simulink model "souk_dual_pipeline_4x2"  (R2021a)
settings: Solver=VariableStepAuto  StartTime=0.0  StopTime=10.0  RelTol=1e-3
undefined symbols (not defined in the file): datetime, posixtime
libraries: xbsIndex_r4 x14695, casper_library_misc x154, casper_library_delays x68, casper_library_reorder x64, casper_library_multipliers x16, xps_library x9
(part 2 of 164)

== system souk_dual_pipeline_4x2/p0 ==
  din_i : Inport
  din_q : Inport  Port=2
  sync_in : Inport  Port=3
  From1 : From  Commented=on  GotoTag=mrst
  Terminator{,1..3} (4 alike) : Terminator
  acc0 : Subsystem  mask{nchan_serial_bits=8, nwindow_bits=11}
      mask prompts: nchan_serial_bits "Number of serial channels (2^?)"; nwindow_bits "Window points (2^?)"
  acc1 : Subsystem  mask{nchan_serial_bits=8, nwindow_bits=11}
      mask prompts: nchan_serial_bits "Number of serial channels (2^?)"; nwindow_bits "Window points (2^?)"
  autocorr : Subsystem  Commented=on  mask{nchan_bits=12-4, npol_bits=0}
      mask prompts: nchan_bits "Number of *SERIAL* channels (2^?)"; npol_bits "Number of pols (2^?)"
  chan_select : Subsystem  mask{serial_chan_bits=8}
      mask prompts: serial_chan_bits "Number of serial channels (2^?)"
  cordic_gen : Subsystem  Commented=on  mask{n_cordic=1, n_parallel=8}
      mask prompts: n_cordic "Number of independent generators?"; n_parallel "Number of parallel samples?"
  edge_detect : casper_library_misc/edge_detect  edge=Rising  polarity=Active High  x_in=[0 0.08 0.08 0.16 0.16 0.24 0.24 0.32 0.32 0.4]  y_in="[-1 -1  1  1  1  1  1  1  1  1]"  x_out=[0.6 0.68 0.68 0.76 0.76 0.84 0.84 0.92 0.92 1]  y_out="[-1 -1  1  1 -1 -1 -1 -1 -1 -1]"
  input : Subsystem
  lut_gen : Subsystem  Commented=on  mask{n_generator=1, n_parallel=8, n_sample_bits=12}
      mask prompts: n_generator "Number of independent generators?"; n_parallel "Number of parallel samples?"; n_sample_bits "Number of samples total (2^?)"
  mix : Subsystem  mask{serial_chan_bits=8}
      mask prompts: serial_chan_bits "Number of serial channels (2^?)"
  output : Subsystem  Commented=on
  pfb : Subsystem
  pfbtvg : Subsystem  Commented=through  mask{serial_chan_bits=8}
      mask prompts: serial_chan_bits "Serial channels (2^?)"
  psb : Subsystem
  pulse_ext : Subsystem  AncestorBlock=casper_library_misc/pulse_ext  mask(pulse_ext){pulse_len=2^25}
      mask prompts: pulse_len "Length of Pulse"
      mask help:
      | Extends a boolean signal to be high for the specified number of clocks after
      | the last high input.
      mask init:
      | bits = ceil(log2(pulse_len+1));
      mask Help: eval('xlWeb(''http://casper.berkeley.edu/wiki/Pulse_ext'')')
  sync : Subsystem  mask{sync_lsb_bits=14, sync_period=307200000}
      mask prompts: sync_lsb_bits "Allowed sync granularity (2^?)"; sync_period "Expected_sync_period"
  synth_input_reorder : Subsystem  mask{serial_chan_bits=8}
      mask prompts: serial_chan_bits "Number of serial channels (2^?)"
  synth_offset_input_reorder : Subsystem  mask{serial_chan_bits=8}
      mask prompts: serial_chan_bits "Number of serial channels (2^?)"
  zoom_acc : Subsystem  Commented=on  mask{nchan_serial_bits=10, downsample_factor=256}
      mask prompts: nchan_serial_bits "Number of serial channels (2^?)"; downsample_factor "Downsample factor?"
  zoom_fft : Subsystem  Commented=on
  dout : Outport
  pps_led : Outport  Port=2
  wires:
  synth_input_reorder/2(dout) -> psb/2(din0)
  chan_select/1(sync_out) -> mix/1(sync), zoom_fft/1(sync)
  zoom_fft/1(sync_out) -> zoom_acc/1(sync)
  synth_offset_input_reorder/2(dout) -> psb/3(din1)
  lut_gen/1(dout) -> output/2(din1)
  cordic_gen/1(dout) -> output/1(din0)
  [sync_gen] -> input/1(sync)
  sync/4(sync_out) -> [sync_gen]
  [mrst] -> mix/3(rst)
  pfbtvg/2(dout) -> chan_select/2(din), autocorr/2(din)
  input/2(dout) -> pfb/2(din)
  sync/1(arm_noise) -> Terminator/1
  chan_select/2(dout) -> mix/2(din), zoom_fft/2(din)
  din_i/1 -> input/2(din_i)
  From1/1 -> autocorr/3(rst)
  sync/7(tt_int_out) -> Terminator2/1
  sync/8(err_out) -> Terminator3/1
  mix/2(dout) -> acc1/2(din), acc0/2(din)
  sync/6(timed_sync) -> Terminator1/1
  pfb/2(dout) -> pfbtvg/2(din)
  mix/3(lo_out) -> synth_offset_input_reorder/2(din), synth_input_reorder/2(din)
  synth_input_reorder/1(sync_out) -> psb/1(sync)
  [mrst] -> acc0/4(rst)
  sync/2(tt_sync) -> [sync_time]
  mix/4(lo_sync_out) -> synth_offset_input_reorder/1(sync), synth_input_reorder/1(sync)
  mix/1(sync_out) -> acc1/1(sync), acc0/1(sync)
  din_q/1 -> input/3(din_q)
  pfb/1(sync_out) -> pfbtvg/1(sync)
  [sync_time] -> acc0/3(tt)
  pfbtvg/1(sync_out) -> chan_select/1(sync), autocorr/1(sync)
  sync/5(mrst) -> [mrst]
  psb/2(dout) -> input/4(dac), dout/1, output/3(din2)
  input/1(sync_out) -> pfb/1(sync)
  [mrst] -> acc1/4(rst)
  zoom_fft/2(dout) -> zoom_acc/2(din)
  [sync_time] -> acc1/3(tt)
  sync_in/1 -> edge_detect/1
  edge_detect/1 -> sync/1(sync_in)
  sync/3(ext_sync) -> pulse_ext/1(in)
  pulse_ext/1(out) -> pps_led/1

== system souk_dual_pipeline_4x2/p1 ==
  din_i : Inport
  din_q : Inport  Port=2
  sync_in : Inport  Port=3
  From1 : From  Commented=on  GotoTag=mrst
  Terminator{,1..3} (4 alike) : Terminator
  acc0 : Subsystem  mask{nchan_serial_bits=8, nwindow_bits=11}
      mask prompts: nchan_serial_bits "Number of serial channels (2^?)"; nwindow_bits "Window points (2^?)"
  acc1 : Subsystem  mask{nchan_serial_bits=8, nwindow_bits=11}
      mask prompts: nchan_serial_bits "Number of serial channels (2^?)"; nwindow_bits "Window points (2^?)"
  autocorr : Subsystem  Commented=on  mask{nchan_bits=12-4, npol_bits=0}
      mask prompts: nchan_bits "Number of *SERIAL* channels (2^?)"; npol_bits "Number of pols (2^?)"
  chan_select : Subsystem  mask{serial_chan_bits=8}
      mask prompts: serial_chan_bits "Number of serial channels (2^?)"
  cordic_gen : Subsystem  Commented=on  mask{n_cordic=1, n_parallel=8}
      mask prompts: n_cordic "Number of independent generators?"; n_parallel "Number of parallel samples?"
  edge_detect : casper_library_misc/edge_detect  edge=Rising  polarity=Active High  x_in=[0 0.08 0.08 0.16 0.16 0.24 0.24 0.32 0.32 0.4]  y_in="[-1 -1  1  1  1  1  1  1  1  1]"  x_out=[0.6 0.68 0.68 0.76 0.76 0.84 0.84 0.92 0.92 1]  y_out="[-1 -1  1  1 -1 -1 -1 -1 -1 -1]"
  input : Subsystem
  lut_gen : Subsystem  Commented=on  mask{n_generator=1, n_parallel=8, n_sample_bits=12}
      mask prompts: n_generator "Number of independent generators?"; n_parallel "Number of parallel samples?"; n_sample_bits "Number of samples total (2^?)"
  mix : Subsystem  mask{serial_chan_bits=8}
      mask prompts: serial_chan_bits "Number of serial channels (2^?)"
  output : Subsystem  Commented=on
  pfb : Subsystem
  pfbtvg : Subsystem  Commented=through  mask{serial_chan_bits=8}
      mask prompts: serial_chan_bits "Serial channels (2^?)"
  psb : Subsystem
  pulse_ext : Subsystem  AncestorBlock=casper_library_misc/pulse_ext  mask(pulse_ext){pulse_len=2^25}
      mask prompts: pulse_len "Length of Pulse"
      mask help:
      | Extends a boolean signal to be high for the specified number of clocks after
      | the last high input.
      mask init:
      | bits = ceil(log2(pulse_len+1));
      mask Help: eval('xlWeb(''http://casper.berkeley.edu/wiki/Pulse_ext'')')
  sync : Subsystem  mask{sync_lsb_bits=14, sync_period=307200000}
      mask prompts: sync_lsb_bits "Allowed sync granularity (2^?)"; sync_period "Expected_sync_period"
  synth_input_reorder : Subsystem  mask{serial_chan_bits=8}
      mask prompts: serial_chan_bits "Number of serial channels (2^?)"
  synth_offset_input_reorder : Subsystem  mask{serial_chan_bits=8}
      mask prompts: serial_chan_bits "Number of serial channels (2^?)"
  zoom_acc : Subsystem  Commented=on  mask{nchan_serial_bits=10, downsample_factor=256}
      mask prompts: nchan_serial_bits "Number of serial channels (2^?)"; downsample_factor "Downsample factor?"
  zoom_fft : Subsystem  Commented=on
  dout : Outport
  pps_led : Outport  Port=2
  wires:
  pulse_ext/1(out) -> pps_led/1
  sync/3(ext_sync) -> pulse_ext/1(in)
  edge_detect/1 -> sync/1(sync_in)
  sync_in/1 -> edge_detect/1
  [sync_time] -> acc1/3(tt)
  zoom_fft/2(dout) -> zoom_acc/2(din)
  [mrst] -> acc1/4(rst)
  input/1(sync_out) -> pfb/1(sync)
  psb/2(dout) -> output/3(din2), dout/1, input/4(dac)
  sync/5(mrst) -> [mrst]
  pfbtvg/1(sync_out) -> autocorr/1(sync), chan_select/1(sync)
  [sync_time] -> acc0/3(tt)
  pfb/1(sync_out) -> pfbtvg/1(sync)
  din_q/1 -> input/3(din_q)
  mix/1(sync_out) -> acc0/1(sync), acc1/1(sync)
  mix/4(lo_sync_out) -> synth_input_reorder/1(sync), synth_offset_input_reorder/1(sync)
  sync/2(tt_sync) -> [sync_time]
  [mrst] -> acc0/4(rst)
  synth_input_reorder/1(sync_out) -> psb/1(sync)
  mix/3(lo_out) -> synth_input_reorder/2(din), synth_offset_input_reorder/2(din)
  pfb/2(dout) -> pfbtvg/2(din)
  sync/6(timed_sync) -> Terminator1/1
  mix/2(dout) -> acc0/2(din), acc1/2(din)
  sync/8(err_out) -> Terminator3/1
  sync/7(tt_int_out) -> Terminator2/1
  From1/1 -> autocorr/3(rst)
  din_i/1 -> input/2(din_i)
  chan_select/2(dout) -> zoom_fft/2(din), mix/2(din)
  sync/1(arm_noise) -> Terminator/1
  input/2(dout) -> pfb/2(din)
  pfbtvg/2(dout) -> autocorr/2(din), chan_select/2(din)
  [mrst] -> mix/3(rst)
  sync/4(sync_out) -> [sync_gen]
  [sync_gen] -> input/1(sync)
  cordic_gen/1(dout) -> output/1(din0)
  lut_gen/1(dout) -> output/2(din1)
  synth_offset_input_reorder/2(dout) -> psb/3(din1)
  zoom_fft/1(sync_out) -> zoom_acc/1(sync)
  chan_select/1(sync_out) -> zoom_fft/1(sync), mix/1(sync)
  synth_input_reorder/2(dout) -> psb/2(din0)

== system souk_dual_pipeline_4x2/rfdc ==
  m00_axis_tdata_sim : Inport
  m01_axis_tdata_sim : Inport  Port=2
  m02_axis_tdata_sim : Inport  Port=3
  m03_axis_tdata_sim : Inport  Port=4
  s00_axis_tdata : Inport  Port=5
  s20_axis_tdata : Inport  Port=6
  souk_dual_pipeline_4x2_rfdc_m00_axis_tdata : xbsIndex_r4/Gateway In  gui_display_data_type=Fixed-point  arith_type="Signed  (2's comp)"  n_bits=128  bin_pt=0  preci_type=Single  exp_width=8  frac_width=24  rows=1  columns=1  quantization="Round  (unbiased: +/- Inf)"  overflow=Saturate  period=1  UseAsADC=off  ADCChannel='1'  interface=None  automatic_assignment=on  address_offset=hex2dec('00')  intf_name=''  intf_description=''  default_value=0  timing_constraint=None  locs_specified=off  LOCs={}  io_standard={}  inherit_from_input=off  hdl_port=on  dbl_ovrd=off  block_type=gatewayin
  souk_dual_pipeline_4x2_rfdc_m01_axis_tdata : xbsIndex_r4/Gateway In  gui_display_data_type=Fixed-point  arith_type="Signed  (2's comp)"  n_bits=128  bin_pt=0  preci_type=Single  exp_width=8  frac_width=24  rows=1  columns=1  quantization="Round  (unbiased: +/- Inf)"  overflow=Saturate  period=1  UseAsADC=off  ADCChannel='1'  interface=None  automatic_assignment=on  address_offset=hex2dec('00')  intf_name=''  intf_description=''  default_value=0  timing_constraint=None  locs_specified=off  LOCs={}  io_standard={}  inherit_from_input=off  hdl_port=on  dbl_ovrd=off  block_type=gatewayin
  souk_dual_pipeline_4x2_rfdc_m02_axis_tdata : xbsIndex_r4/Gateway In  gui_display_data_type=Fixed-point  arith_type="Signed  (2's comp)"  n_bits=128  bin_pt=0  preci_type=Single  exp_width=8  frac_width=24  rows=1  columns=1  quantization="Round  (unbiased: +/- Inf)"  overflow=Saturate  period=1  UseAsADC=off  ADCChannel='1'  interface=None  automatic_assignment=on  address_offset=hex2dec('00')  intf_name=''  intf_description=''  default_value=0  timing_constraint=None  locs_specified=off  LOCs={}  io_standard={}  inherit_from_input=off  hdl_port=on  dbl_ovrd=off  block_type=gatewayin
  souk_dual_pipeline_4x2_rfdc_m03_axis_tdata : xbsIndex_r4/Gateway In  gui_display_data_type=Fixed-point  arith_type="Signed  (2's comp)"  n_bits=128  bin_pt=0  preci_type=Single  exp_width=8  frac_width=24  rows=1  columns=1  quantization="Round  (unbiased: +/- Inf)"  overflow=Saturate  period=1  UseAsADC=off  ADCChannel='1'  interface=None  automatic_assignment=on  address_offset=hex2dec('00')  intf_name=''  intf_description=''  default_value=0  timing_constraint=None  locs_specified=off  LOCs={}  io_standard={}  inherit_from_input=off  hdl_port=on  dbl_ovrd=off  block_type=gatewayin
  souk_dual_pipeline_4x2_rfdc_s00_axis_tdata : xbsIndex_r4/Gateway Out  inherit_from_input=off  hdl_port=on  rows=1  columns=1  UseAsDAC=off  DACChannel='1'  interface=None  automatic_assignment=on  address_offset=hex2dec('00')  intf_name=''  intf_description=''  timing_constraint=None  locs_specified=off  LOCs={}  io_standard={}  block_type=gatewayout
  souk_dual_pipeline_4x2_rfdc_s20_axis_tdata : xbsIndex_r4/Gateway Out  inherit_from_input=off  hdl_port=on  rows=1  columns=1  UseAsDAC=off  DACChannel='1'  interface=None  automatic_assignment=on  address_offset=hex2dec('00')  intf_name=''  intf_description=''  timing_constraint=None  locs_specified=off  LOCs={}  io_standard={}  block_type=gatewayout
  m00_axis_tdata : Outport  VectorParamsAs1DForOutWhenUnconnected=off
  m01_axis_tdata : Outport  Port=2
  m02_axis_tdata : Outport  Port=3  VectorParamsAs1DForOutWhenUnconnected=off
  m03_axis_tdata : Outport  Port=4
  s00_axis_tdata_sim : Outport  Port=5  VectorParamsAs1DForOutWhenUnconnected=off
  s20_axis_tdata_sim : Outport  Port=6
  wires:
  m00_axis_tdata_sim/1 -> souk_dual_pipeline_4x2_rfdc_m00_axis_tdata/1
  souk_dual_pipeline_4x2_rfdc_m00_axis_tdata/1 -> m00_axis_tdata/1  "m00_axis_tdata"
  m01_axis_tdata_sim/1 -> souk_dual_pipeline_4x2_rfdc_m01_axis_tdata/1
  souk_dual_pipeline_4x2_rfdc_m01_axis_tdata/1 -> m01_axis_tdata/1  "m01_axis_tdata"
  m02_axis_tdata_sim/1 -> souk_dual_pipeline_4x2_rfdc_m02_axis_tdata/1
  souk_dual_pipeline_4x2_rfdc_m02_axis_tdata/1 -> m02_axis_tdata/1  "m02_axis_tdata"
  m03_axis_tdata_sim/1 -> souk_dual_pipeline_4x2_rfdc_m03_axis_tdata/1
  souk_dual_pipeline_4x2_rfdc_m03_axis_tdata/1 -> m03_axis_tdata/1  "m03_axis_tdata"
  s00_axis_tdata/1 -> souk_dual_pipeline_4x2_rfdc_s00_axis_tdata/1
  souk_dual_pipeline_4x2_rfdc_s00_axis_tdata/1 -> s00_axis_tdata_sim/1  "s00_axis_tdata_sim"
  s20_axis_tdata/1 -> souk_dual_pipeline_4x2_rfdc_s20_axis_tdata/1
  souk_dual_pipeline_4x2_rfdc_s20_axis_tdata/1 -> s20_axis_tdata_sim/1  "s20_axis_tdata_sim"

== system souk_dual_pipeline_4x2/version ==
  Concat : xbsIndex_r4/Concat  num_inputs=4  dbl_ovrd=off  block_type=concat
  Constant : xbsIndex_r4/Constant  const=ver_maj  gui_display_data_type=Fixed-point  arith_type=Unsigned  n_bits=8  bin_pt=0  preci_type=Single  exp_width=8  frac_width=24  explicit_period=on  period=1  equ=P=C  opselect=C  inp2=PCIN>>17  opr=+  inp1=P  carry=CIN  dbl_ovrd=off  block_type=constant
  Constant1 : xbsIndex_r4/Constant  const=ver_min  gui_display_data_type=Fixed-point  arith_type=Unsigned  n_bits=8  bin_pt=0  preci_type=Single  exp_width=8  frac_width=24  explicit_period=on  period=1  equ=P=C  opselect=C  inp2=PCIN>>17  opr=+  inp1=P  carry=CIN  dbl_ovrd=off  block_type=constant
  Constant2 : xbsIndex_r4/Constant  const=ver_rev  gui_display_data_type=Fixed-point  arith_type=Unsigned  n_bits=8  bin_pt=0  preci_type=Single  exp_width=8  frac_width=24  explicit_period=on  period=1  equ=P=C  opselect=C  inp2=PCIN>>17  opr=+  inp1=P  carry=CIN  dbl_ovrd=off  block_type=constant
  Constant3 : xbsIndex_r4/Constant  const=ver_bugfix  gui_display_data_type=Fixed-point  arith_type=Unsigned  n_bits=8  bin_pt=0  preci_type=Single  exp_width=8  frac_width=24  explicit_period=on  period=1  equ=P=C  opselect=C  inp2=PCIN>>17  opr=+  inp1=P  carry=CIN  dbl_ovrd=off  block_type=constant
  Constant4 : xbsIndex_r4/Constant  const=fw_type  gui_display_data_type=Fixed-point  arith_type=Unsigned  n_bits=32  bin_pt=0  preci_type=Single  exp_width=8  frac_width=24  explicit_period=on  period=1  equ=P=C  opselect=C  inp2=PCIN>>17  opr=+  inp1=P  carry=CIN  dbl_ovrd=off  block_type=constant
  Terminator1 : Terminator
  Terminator2 : Terminator
  timestamp : Subsystem  Tag=xps:sw_reg  AncestorBlock=xps_library/Memory/software_register  mask(swreg){io_dir=To Processor, io_delay=0, init_val=0, sample_period=1, names=reg, bitwidths=32, bin_pts=0, arith_types=0, sim_port=on, show_format=off, desc_str=Unix timestamp, in integer seconds, when firmware was last compiled.}
      mask prompts: io_dir "I/O direction"; io_delay "I/O delay"; init_val "Initial Value"; sample_period "Sample period"; names "Bitfield names [msb...lsb]"; bitwidths "Bitfield widths"; bin_pts "Bitfield binary pts"; arith_types "Bitfield types, ufix=0, fix=1, bool=2"; sim_port "Provide sim input/output?"; show_format "Print format string?"; desc_str "Description"
      mask help: A 32-bit software-accessible register. Can be divided into bitfields of varying widths and types using the fields in the block mask.
      mask init:
      | swreg_init(gcb)
      settings: AttributesFormatString=1output
      mask TypeOptions io_dir: To Processor | From Processor
      mask Callback io_dir:
      | mask_enables = get_param(gcb, 'MaskEnables');
      | mask_names = get_param(gcb, 'MaskNames');
      | if strcmp(get_param(gcb, 'io_dir'), 'To Processor'),
      |     mask_enables{ismember(mask_names, 'sample_period')} = 'off';
      | else
      |     mask_enables{ismember(mask_names, 'sample_period')} = 'on';
      | end
      | set_param(gcb, 'MaskEnables', mask_enables);
      mask DialogControl ParameterTabVar0 Prompt: Setup
  type : Subsystem  Tag=xps:sw_reg  AncestorBlock=xps_library/Memory/software_register  mask(swreg){io_dir=To Processor, io_delay=0, init_val=0, sample_period=1, names=reg, bitwidths=32, bin_pts=0, arith_types=0, sim_port=on, show_format=off, desc_str=|}
      mask desc_str=|
      | Version register. Bits ``[31:24]``: major version. Bits
      | ``[23:16]``: minor version. Bits ``[15:8]``: revision.
      | Bits ``[7:0]``: buxfix.
      mask prompts: io_dir "I/O direction"; io_delay "I/O delay"; init_val "Initial Value"; sample_period "Sample period"; names "Bitfield names [msb...lsb]"; bitwidths "Bitfield widths"; bin_pts "Bitfield binary pts"; arith_types "Bitfield types, ufix=0, fix=1, bool=2"; sim_port "Provide sim input/output?"; show_format "Print format string?"; desc_str "Description"
      mask help: A 32-bit software-accessible register. Can be divided into bitfields of varying widths and types using the fields in the block mask.
      mask init:
      | swreg_init(gcb)
      settings: AttributesFormatString=1output
      mask TypeOptions io_dir: To Processor | From Processor
      mask Callback io_dir:
      | mask_enables = get_param(gcb, 'MaskEnables');
      | mask_names = get_param(gcb, 'MaskNames');
      | if strcmp(get_param(gcb, 'io_dir'), 'To Processor'),
      |     mask_enables{ismember(mask_names, 'sample_period')} = 'off';
      | else
      |     mask_enables{ismember(mask_names, 'sample_period')} = 'on';
      | end
      | set_param(gcb, 'MaskEnables', mask_enables);
      mask DialogControl ParameterTabVar0 Prompt: Setup
  unix_time : xbsIndex_r4/Constant  const=round(posixtime(datetime('now')))  gui_display_data_type=Fixed-point  arith_type=Unsigned  n_bits=32  bin_pt=0  preci_type=Single  exp_width=8  frac_width=24  explicit_period=on  period=1  equ=P=C  opselect=C  inp2=PCIN>>17  opr=+  inp1=P  carry=CIN  dbl_ovrd=off  block_type=constant
  version : Subsystem  Tag=xps:sw_reg  AncestorBlock=xps_library/Memory/software_register  mask(swreg){io_dir=To Processor, io_delay=0, init_val=0, sample_period=1, names=reg, bitwidths=32, bin_pts=0, arith_types=0, sim_port=on, show_format=off, desc_str=|}
      mask desc_str=|
      | Version register. Bits ``[31:24]``: major version. Bits
      | ``[23:16]``: minor version. Bits ``[15:8]``: revision.
      | Bits ``[7:0]``: buxfix.
      mask prompts: io_dir "I/O direction"; io_delay "I/O delay"; init_val "Initial Value"; sample_period "Sample period"; names "Bitfield names [msb...lsb]"; bitwidths "Bitfield widths"; bin_pts "Bitfield binary pts"; arith_types "Bitfield types, ufix=0, fix=1, bool=2"; sim_port "Provide sim input/output?"; show_format "Print format string?"; desc_str "Description"
      mask help: A 32-bit software-accessible register. Can be divided into bitfields of varying widths and types using the fields in the block mask.
      mask init:
      | swreg_init(gcb)
      settings: AttributesFormatString=1output
      mask TypeOptions io_dir: To Processor | From Processor
      mask Callback io_dir:
      | mask_enables = get_param(gcb, 'MaskEnables');
      | mask_names = get_param(gcb, 'MaskNames');
      | if strcmp(get_param(gcb, 'io_dir'), 'To Processor'),
      |     mask_enables{ismember(mask_names, 'sample_period')} = 'off';
      | else
      |     mask_enables{ismember(mask_names, 'sample_period')} = 'on';
      | end
      | set_param(gcb, 'MaskEnables', mask_enables);
      mask DialogControl ParameterTabVar0 Prompt: Setup
  tag scope: ver_maj=global
  wires:
  Constant1/1 -> Concat/2
  Concat/1 -> version/1(out_reg)
  Constant/1 -> [ver_maj], Concat/1
  version/1(sim_out_reg) -> Terminator1/1
  Constant2/1 -> Concat/3
  Constant3/1 -> Concat/4
  timestamp/1(sim_out_reg) -> Terminator2/1
  unix_time/1 -> timestamp/1(out_reg)
  Constant4/1 -> type/1(out_reg)
